simulink model "TosAeb"  (R2021a)
settings: Solver=FixedStepDiscrete  StartTime=0.0  StopTime=inf  FixedStep=0.01  RelTol=1e-3
undefined symbols (not defined in the file or in its folder): ACCModSts, AccSts1, AEB_ObjectMovingOrientation, AEB_ObjectMovingState, CN_BTCActive, CN_CurrentGearR, CN_CurrentGearR_DHT, CN_PTCActive, CN_VDCActive, CN_VSC_EspOff, CN_VSC_FCW_AEB_Error, CN_VSC_FCW_AEB_NoDispay, CN_VSC_FCW_AEB_PerfmDgdtn, CN_VSC_FCW_AEB_TempUnAvlbl, CN_VSC_VehStandstill, CNaccelAvoidMax, CNaccelAvoidMin, CNbrakePedalPress, CNcomfortStrLeftAccelMax, CNcomfortStrLeftAccelMin, CNcomfortStrRightAccelMax, CNcomfortStrRightAccelMin, CNDrvDoorOpen, CNDrvModeEco, CNDrvModeNorm, CNDrvModeOther, CNDrvModeSport, CNDrvSBRreminder, CNNull, CNobjInvalidID, CNPassDoorOpen, CNPassSBRreminder, CNPEBSAccelNegInf, CNPEBSAccelNull, CNPEBSTimeInf, CNPEBSTimeNull, CNSTOPMAX, CNSTOPNULL, CNsubjWidth, CNSwtOFF, CNSwtON, CNTTCMAX, CNTTCNULL, CNVehConfigAEBCycNone, CNVehConfigAEBNone, CNVehConfigAEBPedNone, CNVehConfigFCWNone, CNVehConfigJANone, CollisionSeverity, FALSE, FCWSnvty, JACycObjCalcInfoDefaultParams, JAPedObjCalcInfoDefaultParams, JAVehObjCalcInfoDefaultParams, latCycObjCalcInfoDefaultParams, latPedObjCalcInfoDefaultParams, longObjCalcInfoDefaultParams, ModSts, movingStatus, ObjectType ... (+1112)
libraries: simulink x3031, AEBSFunctionLib x50, Ta_Library x33, AEBSStateMachineLib x23, Mylibrary x12, simulink_extras x7, ObjSelect_lib x3, dspstat3 x2
(part 31 of 102)

== system TosAeb/FcwAebJa_Step_sys/ALG_AEBSystem/ALG_AEBController/AEB_StateMachineCalcApp/JA_AEBStateMachine/JA_pedAEBfullStateMachine/ActivationCondCalc ==
  JA_pedAEBfullSuppCond : Inport
  JA_pedAEBfullSuppArb : Inport  Port=2
  JA_pedAEBfullFuncImpSuppCond : Inport  Port=3
  Compare To Constant1 : simulink/Logic and Bit Operations/Compare To Constant  relop===  const=FALSE  OutDataTypeStr=boolean
  Compare To Constant2 : simulink/Logic and Bit Operations/Compare To Constant  relop===  const=FALSE  OutDataTypeStr=boolean
  Compare To Constant3 : simulink/Logic and Bit Operations/Compare To Constant  relop===  const=FALSE  OutDataTypeStr=boolean
  Logical Operator : Logic  Inputs=3  AllPortsSameDT=off  OutDataTypeStr=boolean
  ActivationCondFulfilled : Outport
  wires:
  JA_pedAEBfullSuppCond/1 -> Compare To Constant3/1
  JA_pedAEBfullSuppArb/1 -> Compare To Constant1/1
  JA_pedAEBfullFuncImpSuppCond/1 -> Compare To Constant2/1
  Compare To Constant1/1 -> Logical Operator/2
  Compare To Constant3/1 -> Logical Operator/1
  Compare To Constant2/1 -> Logical Operator/3
  Logical Operator/1 -> ActivationCondFulfilled/1

== system TosAeb/FcwAebJa_Step_sys/ALG_AEBSystem/ALG_AEBController/AEB_StateMachineCalcApp/JA_AEBStateMachine/JA_pedAEBfullStateMachine/ActiveStateAborCondCalc ==
  JA_pedAEBfullAborCond : Inport
  JA_pedAEBfullAborArb : Inport  Port=2
  JA_pedAEBfullFuncImpAborCond : Inport  Port=3
  JABrkManeuAborCond : Inport  Port=4
  Compare To Constant{,1..3} (4 alike) : simulink/Logic and Bit Operations/Compare To Constant  relop===  const=TRUE  OutDataTypeStr=boolean
  Logical Operator : Logic  Operator=OR  Inputs=4  AllPortsSameDT=off  OutDataTypeStr=boolean
  ActiveStateAborCondFulfilled : Outport
  wires:
  Logical Operator/1 -> ActiveStateAborCondFulfilled/1
  Compare To Constant2/1 -> Logical Operator/3
  Compare To Constant/1 -> Logical Operator/1
  Compare To Constant1/1 -> Logical Operator/2
  JA_pedAEBfullFuncImpAborCond/1 -> Compare To Constant2/1
  JA_pedAEBfullAborArb/1 -> Compare To Constant1/1
  JA_pedAEBfullAborCond/1 -> Compare To Constant/1
  JABrkManeuAborCond/1 -> Compare To Constant3/1
  Compare To Constant3/1 -> Logical Operator/4

== system TosAeb/FcwAebJa_Step_sys/ALG_AEBSystem/ALG_AEBController/AEB_StateMachineCalcApp/JA_AEBStateMachine/JA_pedAEBfullStateMachine/StandstillStateAborCondCalc ==
  JA_pedAEBfullAborCond : Inport
  JA_pedAEBfullAborArb : Inport  Port=2
  JA_pedAEBfullFuncImpAborCond : Inport  Port=3
  JABrkManeuAborCond : Inport  Port=4
  Compare To Constant{,1..3} (4 alike) : simulink/Logic and Bit Operations/Compare To Constant  relop===  const=TRUE  OutDataTypeStr=boolean
  Logical Operator : Logic  Operator=OR  Inputs=4  AllPortsSameDT=off  OutDataTypeStr=boolean
  ActiveStateAborCondFulfilled : Outport
  wires:
  Compare To Constant3/1 -> Logical Operator/4
  JABrkManeuAborCond/1 -> Compare To Constant3/1
  JA_pedAEBfullAborCond/1 -> Compare To Constant/1
  JA_pedAEBfullAborArb/1 -> Compare To Constant1/1
  JA_pedAEBfullFuncImpAborCond/1 -> Compare To Constant2/1
  Compare To Constant1/1 -> Logical Operator/2
  Compare To Constant/1 -> Logical Operator/1
  Compare To Constant2/1 -> Logical Operator/3
  Logical Operator/1 -> ActiveStateAborCondFulfilled/1

== system TosAeb/FcwAebJa_Step_sys/ALG_AEBSystem/ALG_AEBController/AEB_StateMachineCalcApp/JA_AEBStateMachine/JA_pedPrefillStateMachine/AEBFuncFaultMap ==
  FCW_AEB_VehFctSts : Inport
  Compare To Constant : simulink/Logic and Bit Operations/Compare To Constant  relop===  const=CN_VSC_FCW_AEB_Error  OutDataTypeStr=boolean
  Fault : Outport
  wires:
  FCW_AEB_VehFctSts/1 -> Compare To Constant/1
  Compare To Constant/1 -> Fault/1

== system TosAeb/FcwAebJa_Step_sys/ALG_AEBSystem/ALG_AEBController/AEB_StateMachineCalcApp/JA_AEBStateMachine/JA_pedPrefillStateMachine/ActivationCondCalc ==
  JA_pedPrefillSuppCond : Inport
  JA_pedPrefillSuppArb : Inport  Port=2
  JA_pedPrefillFuncImpSuppCond : Inport  Port=3
  Compare To Constant1 : simulink/Logic and Bit Operations/Compare To Constant  relop===  const=FALSE  OutDataTypeStr=boolean
  Compare To Constant2 : simulink/Logic and Bit Operations/Compare To Constant  relop===  const=FALSE  OutDataTypeStr=boolean
  Compare To Constant3 : simulink/Logic and Bit Operations/Compare To Constant  relop===  const=FALSE  OutDataTypeStr=boolean
  Logical Operator : Logic  Inputs=3  AllPortsSameDT=off  OutDataTypeStr=boolean
  ActivationCondFulfilled : Outport
  wires:
  JA_pedPrefillSuppCond/1 -> Compare To Constant3/1
  JA_pedPrefillSuppArb/1 -> Compare To Constant1/1
  JA_pedPrefillFuncImpSuppCond/1 -> Compare To Constant2/1
  Compare To Constant1/1 -> Logical Operator/2
  Compare To Constant3/1 -> Logical Operator/1
  Compare To Constant2/1 -> Logical Operator/3
  Logical Operator/1 -> ActivationCondFulfilled/1

== system TosAeb/FcwAebJa_Step_sys/ALG_AEBSystem/ALG_AEBController/AEB_StateMachineCalcApp/JA_AEBStateMachine/JA_pedPrefillStateMachine/ActiveStateAborCondCalc ==
  JA_pedPrefillAborCond : Inport
  JA_pedPrefillAborArb : Inport  Port=2
  JA_pedPrefillFuncImpAborCond : Inport  Port=3
  ABPManeuAborCond : Inport  Port=4
  Compare To Constant{,1..3} (4 alike) : simulink/Logic and Bit Operations/Compare To Constant  relop===  const=TRUE  OutDataTypeStr=boolean
  Logical Operator : Logic  Operator=OR  Inputs=4  AllPortsSameDT=off  OutDataTypeStr=boolean
  ActiveStateAborCondFulfilled : Outport
  wires:
  Logical Operator/1 -> ActiveStateAborCondFulfilled/1
  Compare To Constant2/1 -> Logical Operator/3
  Compare To Constant/1 -> Logical Operator/1
  Compare To Constant1/1 -> Logical Operator/2
  JA_pedPrefillFuncImpAborCond/1 -> Compare To Constant2/1
  JA_pedPrefillAborArb/1 -> Compare To Constant1/1
  JA_pedPrefillAborCond/1 -> Compare To Constant/1
  ABPManeuAborCond/1 -> Compare To Constant3/1
  Compare To Constant3/1 -> Logical Operator/4

== system TosAeb/FcwAebJa_Step_sys/ALG_AEBSystem/ALG_AEBController/AEB_StateMachineCalcApp/JA_AEBStateMachine/JA_vehAEBStateMachine/AEBFuncFaultMap ==
  FCW_AEB_VehFctSts : Inport
  Compare To Constant : simulink/Logic and Bit Operations/Compare To Constant  relop===  const=CN_VSC_FCW_AEB_Error  OutDataTypeStr=boolean
  Fault : Outport
  wires:
  FCW_AEB_VehFctSts/1 -> Compare To Constant/1
  Compare To Constant/1 -> Fault/1

== system TosAeb/FcwAebJa_Step_sys/ALG_AEBSystem/ALG_AEBController/AEB_StateMachineCalcApp/JA_AEBStateMachine/JA_vehAEBStateMachine/ActivationCondCalc ==
  JA_vehAEBfullSuppCond : Inport
  JA_vehAEBfullSuppArb : Inport  Port=2
  JA_vehAEBfullFuncImpSuppCond : Inport  Port=3
  Compare To Constant1 : simulink/Logic and Bit Operations/Compare To Constant  relop===  const=FALSE  OutDataTypeStr=boolean
  Compare To Constant2 : simulink/Logic and Bit Operations/Compare To Constant  relop===  const=FALSE  OutDataTypeStr=boolean
  Compare To Constant3 : simulink/Logic and Bit Operations/Compare To Constant  relop===  const=FALSE  OutDataTypeStr=boolean
  Logical Operator : Logic  Inputs=3  AllPortsSameDT=off  OutDataTypeStr=boolean
  ActivationCondFulfilled : Outport
  wires:
  JA_vehAEBfullSuppCond/1 -> Compare To Constant3/1
  JA_vehAEBfullSuppArb/1 -> Compare To Constant1/1
  JA_vehAEBfullFuncImpSuppCond/1 -> Compare To Constant2/1
  Compare To Constant1/1 -> Logical Operator/2
  Compare To Constant3/1 -> Logical Operator/1
  Compare To Constant2/1 -> Logical Operator/3
  Logical Operator/1 -> ActivationCondFulfilled/1

== system TosAeb/FcwAebJa_Step_sys/ALG_AEBSystem/ALG_AEBController/AEB_StateMachineCalcApp/JA_AEBStateMachine/JA_vehAEBStateMachine/ActiveStateAborCondCalc ==
  JA_vehAEBfullAborCondNoStandstill : Inport
  JA_vehAEBfullAborArb : Inport  Port=2
  JA_vehAEBfullFuncImpAborCond : Inport  Port=3
  JABrkManeuAborCond : Inport  Port=4
  Compare To Constant{,1..3} (4 alike) : simulink/Logic and Bit Operations/Compare To Constant  relop===  const=TRUE  OutDataTypeStr=boolean
  Logical Operator : Logic  Operator=OR  Inputs=4  AllPortsSameDT=off  OutDataTypeStr=boolean
  ActiveStateAborCondFulfilled : Outport
  wires:
  Logical Operator/1 -> ActiveStateAborCondFulfilled/1
  Compare To Constant2/1 -> Logical Operator/3
  Compare To Constant/1 -> Logical Operator/1
  Compare To Constant1/1 -> Logical Operator/2
  JA_vehAEBfullFuncImpAborCond/1 -> Compare To Constant2/1
  JA_vehAEBfullAborArb/1 -> Compare To Constant1/1
  JA_vehAEBfullAborCondNoStandstill/1 -> Compare To Constant/1
  JABrkManeuAborCond/1 -> Compare To Constant3/1
  Compare To Constant3/1 -> Logical Operator/4

== system TosAeb/FcwAebJa_Step_sys/ALG_AEBSystem/ALG_AEBController/AEB_StateMachineCalcApp/JA_AEBStateMachine/JA_vehAEBStateMachine/StandstillStateAborCondCalc ==
  JA_vehAEBfullAborCondStandstill : Inport
  JA_vehAEBfullAborArb : Inport  Port=2
  JA_vehAEBfullFuncImpAborCond : Inport  Port=3
  JABrkManeuAborCond : Inport  Port=4
  Compare To Constant{,1..3} (4 alike) : simulink/Logic and Bit Operations/Compare To Constant  relop===  const=TRUE  OutDataTypeStr=boolean
  Logical Operator : Logic  Operator=OR  Inputs=4  AllPortsSameDT=off  OutDataTypeStr=boolean
  ActiveStateAborCondFulfilled : Outport
  wires:
  Compare To Constant3/1 -> Logical Operator/4
  JABrkManeuAborCond/1 -> Compare To Constant3/1
  JA_vehAEBfullAborCondStandstill/1 -> Compare To Constant/1
  JA_vehAEBfullAborArb/1 -> Compare To Constant1/1
  JA_vehAEBfullFuncImpAborCond/1 -> Compare To Constant2/1
  Compare To Constant1/1 -> Logical Operator/2
  Compare To Constant/1 -> Logical Operator/1
  Compare To Constant2/1 -> Logical Operator/3
  Logical Operator/1 -> ActiveStateAborCondFulfilled/1

== system TosAeb/FcwAebJa_Step_sys/ALG_AEBSystem/ALG_AEBController/AEB_StateMachineCalcApp/JA_AEBStateMachine/JA_vehPrefillStateMachine/AEBFuncFaultMap ==
  FCW_AEB_VehFctSts : Inport
  Compare To Constant : simulink/Logic and Bit Operations/Compare To Constant  relop===  const=CN_VSC_FCW_AEB_Error  OutDataTypeStr=boolean
  Fault : Outport
  wires:
  FCW_AEB_VehFctSts/1 -> Compare To Constant/1
  Compare To Constant/1 -> Fault/1

== system TosAeb/FcwAebJa_Step_sys/ALG_AEBSystem/ALG_AEBController/AEB_StateMachineCalcApp/JA_AEBStateMachine/JA_vehPrefillStateMachine/ActivationCondCalc ==
  JA_vehPrefillSuppCond : Inport
  JA_vehPrefillSuppArb : Inport  Port=2
  JA_vehPrefillFuncImpSuppCond : Inport  Port=3
  Compare To Constant1 : simulink/Logic and Bit Operations/Compare To Constant  relop===  const=FALSE  OutDataTypeStr=boolean
  Compare To Constant2 : simulink/Logic and Bit Operations/Compare To Constant  relop===  const=FALSE  OutDataTypeStr=boolean
  Compare To Constant3 : simulink/Logic and Bit Operations/Compare To Constant  relop===  const=FALSE  OutDataTypeStr=boolean
  Logical Operator : Logic  Inputs=3  AllPortsSameDT=off  OutDataTypeStr=boolean
  ActivationCondFulfilled : Outport
  wires:
  JA_vehPrefillSuppCond/1 -> Compare To Constant3/1
  JA_vehPrefillSuppArb/1 -> Compare To Constant1/1
  JA_vehPrefillFuncImpSuppCond/1 -> Compare To Constant2/1
  Compare To Constant1/1 -> Logical Operator/2
  Compare To Constant3/1 -> Logical Operator/1
  Compare To Constant2/1 -> Logical Operator/3
  Logical Operator/1 -> ActivationCondFulfilled/1

== system TosAeb/FcwAebJa_Step_sys/ALG_AEBSystem/ALG_AEBController/AEB_StateMachineCalcApp/JA_AEBStateMachine/JA_vehPrefillStateMachine/ActiveStateAborCondCalc ==
  JA_vehPrefillAborCond : Inport
  JA_vehPrefillAborArb : Inport  Port=2
  JA_vehPrefillFuncImpAborCond : Inport  Port=3
  ABPManeuAborCond : Inport  Port=4
  Compare To Constant{,1..3} (4 alike) : simulink/Logic and Bit Operations/Compare To Constant  relop===  const=TRUE  OutDataTypeStr=boolean
  Logical Operator : Logic  Operator=OR  Inputs=4  AllPortsSameDT=off  OutDataTypeStr=boolean
  ActiveStateAborCondFulfilled : Outport
  wires:
  Logical Operator/1 -> ActiveStateAborCondFulfilled/1
  Compare To Constant2/1 -> Logical Operator/3
  Compare To Constant/1 -> Logical Operator/1
  Compare To Constant1/1 -> Logical Operator/2
  JA_vehPrefillFuncImpAborCond/1 -> Compare To Constant2/1
  JA_vehPrefillAborArb/1 -> Compare To Constant1/1
  JA_vehPrefillAborCond/1 -> Compare To Constant/1
  ABPManeuAborCond/1 -> Compare To Constant3/1
  Compare To Constant3/1 -> Logical Operator/4

== system TosAeb/FcwAebJa_Step_sys/ALG_AEBSystem/ALG_AEBController/AEB_StateMachineCalcApp/JaFctStsArb/BrkArb/BrkAborArb ==
  JA_vehAEBModSts : Inport
  JA_pedAEBModSts : Inport  Port=2
  JA_cycAEBModSts : Inport  Port=3
  JA_PTW_AEBModSts : Inport  Port=4
  Bus Creator : BusCreator  Inputs=4
  Bus Selector : BusSelector  OutputSignals=JA_pedAEBModSts,JA_cycAEBModSts,JA_PTW_AEBModSts
  Bus Selector1 : BusSelector  OutputSignals=JA_cycAEBModSts,JA_pedAEBModSts
  Bus Selector2 : BusSelector  OutputSignals=JA_pedAEBModSts
  Compare To Constant{1..2,4..6,12} (6 alike) : simulink/Logic and Bit Operations/Compare To Constant  relop===  const=ModSts.ActiveState  OutDataTypeStr=boolean
  Constant : Constant  Value=FALSE
  Logical Operator : Logic  Operator=OR  Inputs=3  AllPortsSameDT=off  OutDataTypeStr=boolean
  Logical Operator1 : Logic  Operator=OR  AllPortsSameDT=off  OutDataTypeStr=boolean
  JA_vehAEBAborArb : Outport
  JA_PTW_AEBAborArb : Outport  Port=2
  JA_cycAEBAborArb : Outport  Port=3
  JA_pedAEBAborArb : Outport  Port=4
  wires:
  JA_PTW_AEBModSts/1 -> Bus Creator/4  "JA_PTW_AEBModSts"
  JA_cycAEBModSts/1 -> Bus Creator/3  "JA_cycAEBModSts"
  JA_pedAEBModSts/1 -> Bus Creator/2  "JA_pedAEBModSts"
  JA_vehAEBModSts/1 -> Bus Creator/1  "JA_vehAEBModSts"
  Bus Selector/3 -> Compare To Constant1/1  "<JA_PTW_AEBModSts>"
  Bus Selector1/1 -> Compare To Constant5/1  "<JA_cycAEBModSts>"
  Bus Selector1/2 -> Compare To Constant4/1  "<JA_pedAEBModSts>"
  Compare To Constant2/1 -> Logical Operator/2
  Compare To Constant4/1 -> Logical Operator1/2
  Compare To Constant1/1 -> Logical Operator/3
  Bus Selector2/1 -> Compare To Constant12/1  "<JA_pedAEBModSts>"
  Bus Creator/1 -> Bus Selector2/1, Bus Selector1/1, Bus Selector/1
  Compare To Constant6/1 -> Logical Operator/1
  Bus Selector/1 -> Compare To Constant6/1  "<JA_pedAEBModSts>"
  Compare To Constant12/1 -> JA_cycAEBAborArb/1
  Bus Selector/2 -> Compare To Constant2/1  "<JA_cycAEBModSts>"
  Compare To Constant5/1 -> Logical Operator1/1
  Logical Operator/1 -> JA_vehAEBAborArb/1
  Logical Operator1/1 -> JA_PTW_AEBAborArb/1
  Constant/1 -> JA_pedAEBAborArb/1

== system TosAeb/FcwAebJa_Step_sys/ALG_AEBSystem/ALG_AEBController/AEB_StateMachineCalcApp/JaFctStsArb/BrkArb/JA_BrkActvArb ==
  JA_vehAEBModStsArb : Inport
  JA_PTW_AEBModStsArb : Inport  Port=2
  JA_cycAEBModStsArb : Inport  Port=3
  JA_pedAEBModStsArb : Inport  Port=4
  Compare To Constant{,1..3} (4 alike) : simulink/Logic and Bit Operations/Compare To Constant  relop===  const=ModSts.ActiveState  OutDataTypeStr=boolean
  Logical Operator : Logic  Operator=OR  Inputs=4  AllPortsSameDT=off  OutDataTypeStr=boolean
  JA_BrkActvCond : Outport
  wires:
  Compare To Constant2/1 -> Logical Operator/3
  Compare To Constant1/1 -> Logical Operator/2
  Compare To Constant/1 -> Logical Operator/1
  Logical Operator/1 -> JA_BrkActvCond/1
  JA_vehAEBModStsArb/1 -> Compare To Constant/1
  JA_PTW_AEBModStsArb/1 -> Compare To Constant1/1
  JA_cycAEBModStsArb/1 -> Compare To Constant2/1
  JA_pedAEBModStsArb/1 -> Compare To Constant3/1
  Compare To Constant3/1 -> Logical Operator/4

== system TosAeb/FcwAebJa_Step_sys/ALG_AEBSystem/ALG_AEBController/AEB_StateMachineCalcApp/JaFctStsArb/BrkArb/JA_BrkModStsArbtrtn ==
  JA_vehAEBModSts : Inport
  JA_PTW_AEBModSts : Inport  Port=2
  JA_cycAEBModSts : Inport  Port=3
  JA_pedAEBModSts : Inport  Port=4
  JA_vehAEBAborArb : Inport  Port=5
  JA_PTW_AEBAborArb : Inport  Port=6
  JA_cycAEBAborArb : Inport  Port=7
  JA_pedAEBAborArb : Inport  Port=8
  Compare To Constant{,2,4,6} (4 alike) : simulink/Logic and Bit Operations/Compare To Constant  relop=~=  const=ModSts.ActiveState  OutDataTypeStr=boolean
  Compare To Constant{1,3,5,7} (4 alike) : simulink/Logic and Bit Operations/Compare To Constant  relop===  const=FALSE  OutDataTypeStr=boolean
  Constant{,1..3} (4 alike) : Constant  Value=ModSts.StandbyState
  Switch{,1..7} (8 alike) : Switch  Criteria=u2 ~= 0
  JA_vehAEBModStsArb : Outport
  JA_PTW_AEBModStsArb : Outport  Port=2
  JA_cycAEBModStsArb : Outport  Port=3
  JA_pedAEBModStsArb : Outport  Port=4
  wires:
  Compare To Constant/1 -> Switch/2
  Switch1/1 -> Switch/3
  Compare To Constant1/1 -> Switch1/2
  JA_vehAEBAborArb/1 -> Compare To Constant1/1
  Constant/1 -> Switch1/3
  Switch/1 -> JA_vehAEBModStsArb/1
  Compare To Constant2/1 -> Switch2/2
  JA_PTW_AEBAborArb/1 -> Compare To Constant3/1
  Compare To Constant3/1 -> Switch3/2
  Constant1/1 -> Switch3/3
  Switch3/1 -> Switch2/3
  Switch2/1 -> JA_PTW_AEBModStsArb/1
  Switch4/1 -> JA_cycAEBModStsArb/1
  Switch5/1 -> Switch4/3
  Constant2/1 -> Switch5/3
  Compare To Constant5/1 -> Switch5/2
  JA_cycAEBAborArb/1 -> Compare To Constant5/1
  Compare To Constant4/1 -> Switch4/2
  JA_PTW_AEBModSts/1 -> Switch3/1, Switch2/1, Compare To Constant2/1  "JA_PTW_AEBModSts"
  JA_cycAEBModSts/1 -> Compare To Constant4/1, Switch4/1, Switch5/1  "JA_cycAEBModSts"
  JA_vehAEBModSts/1 -> Switch/1, Switch1/1, Compare To Constant/1  "JA_vehAEBModSts"
  JA_pedAEBModSts/1 -> Switch7/1, Switch6/1, Compare To Constant6/1
  Compare To Constant6/1 -> Switch6/2
  JA_pedAEBAborArb/1 -> Compare To Constant7/1
  Compare To Constant7/1 -> Switch7/2
  Constant3/1 -> Switch7/3
  Switch7/1 -> Switch6/3
  Switch6/1 -> JA_pedAEBModStsArb/1

== system TosAeb/FcwAebJa_Step_sys/ALG_AEBSystem/ALG_AEBController/AEB_StateMachineCalcApp/JaFctStsArb/BrkArb/JA_BrkSuppArb ==
  JA_vehAEBModSts : Inport
  JA_pedAEBModSts : Inport  Port=2
  JA_cycAEBModSts : Inport  Port=3
  JA_PTW_AEBModSts : Inport  Port=4
  Bus Creator : BusCreator  Inputs=4
  Bus Selector : BusSelector  OutputSignals=JA_pedAEBModSts,JA_cycAEBModSts,JA_PTW_AEBModSts
  Bus Selector1 : BusSelector  OutputSignals=JA_cycAEBModSts,JA_pedAEBModSts
  Bus Selector2 : BusSelector  OutputSignals=JA_cycAEBModSts
  Compare To Constant{1..2,4..6,12} (6 alike) : simulink/Logic and Bit Operations/Compare To Constant  relop===  const=ModSts.ActiveState  OutDataTypeStr=boolean
  Constant : Constant  Value=FALSE
  Logical Operator : Logic  Operator=OR  Inputs=3  AllPortsSameDT=off  OutDataTypeStr=boolean
  Logical Operator1 : Logic  Operator=OR  AllPortsSameDT=off  OutDataTypeStr=boolean
  JA_vehAEBSuppArb : Outport
  JA_PTW_AEBSuppArb : Outport  Port=2
  JA_cycAEBSuppArb : Outport  Port=3
  JA_pedAEBSuppArb : Outport  Port=4
  wires:
  Logical Operator/1 -> JA_vehAEBSuppArb/1
  Compare To Constant6/1 -> Logical Operator/1
  Compare To Constant2/1 -> Logical Operator/2
  Bus Selector/1 -> Compare To Constant6/1  "<JA_pedAEBModSts>"
  Bus Selector/2 -> Compare To Constant2/1  "<JA_cycAEBModSts>"
  Bus Selector/3 -> Compare To Constant1/1  "<JA_PTW_AEBModSts>"
  Compare To Constant1/1 -> Logical Operator/3
  Logical Operator1/1 -> JA_PTW_AEBSuppArb/1
  Compare To Constant12/1 -> JA_cycAEBSuppArb/1
  Constant/1 -> JA_pedAEBSuppArb/1
  JA_vehAEBModSts/1 -> Bus Creator/1  "JA_vehAEBModSts"
  JA_pedAEBModSts/1 -> Bus Creator/2  "JA_pedAEBModSts"
  JA_cycAEBModSts/1 -> Bus Creator/3  "JA_cycAEBModSts"
  JA_PTW_AEBModSts/1 -> Bus Creator/4  "JA_PTW_AEBModSts"
  Bus Creator/1 -> Bus Selector2/1, Bus Selector1/1, Bus Selector/1
  Bus Selector1/1 -> Compare To Constant5/1  "<JA_cycAEBModSts>"
  Bus Selector1/2 -> Compare To Constant4/1  "<JA_pedAEBModSts>"
  Compare To Constant5/1 -> Logical Operator1/1
  Compare To Constant4/1 -> Logical Operator1/2
  Bus Selector2/1 -> Compare To Constant12/1  "<JA_cycAEBModSts>"

== system TosAeb/FcwAebJa_Step_sys/ALG_AEBSystem/ALG_AEBController/AEB_StateMachineCalcApp/JaFctStsArb/BrkReadyArb/BrkAborArb ==
  JA_vehPrefillModSts : Inport
  JA_pedPrefillModSts : Inport  Port=2
  JA_cycPrefillModSts : Inport  Port=3
  JA_PTW_PrefillModSts : Inport  Port=4
  JA_BrkActvCond : Inport  Port=5
  Bus Creator : BusCreator  Inputs=5
  Bus Selector : BusSelector  OutputSignals=JA_pedAEBModSts,JA_cycAEBModSts,JA_PTW_AEBModSts
  Bus Selector1 : BusSelector  OutputSignals=JA_cycAEBModSts,JA_pedAEBModSts
  Bus Selector2 : BusSelector  OutputSignals=JA_pedAEBModSts
  Compare To Constant{1..2,4..6,12} (6 alike) : simulink/Logic and Bit Operations/Compare To Constant  relop===  const=ModSts.ActiveState  OutDataTypeStr=boolean
  Constant : Constant  Value=FALSE
  Logical Operator : Logic  Operator=OR  Inputs=3  AllPortsSameDT=off  OutDataTypeStr=boolean
  Logical Operator1 : Logic  Operator=OR  AllPortsSameDT=off  OutDataTypeStr=boolean
  JA_vehPrefillAborArb : Outport
  JA_PTW_PrefillAborArb : Outport  Port=2
  JA_cycPrefillAborArb : Outport  Port=3
  JA_pedPrefillAborArb : Outport  Port=4
  wires:
  Constant/1 -> JA_pedPrefillAborArb/1
  Logical Operator1/1 -> JA_PTW_PrefillAborArb/1
  Logical Operator/1 -> JA_vehPrefillAborArb/1
  Compare To Constant5/1 -> Logical Operator1/1
  Bus Selector/2 -> Compare To Constant2/1  "<JA_cycAEBModSts>"
  Compare To Constant12/1 -> JA_cycPrefillAborArb/1
  Bus Selector/1 -> Compare To Constant6/1  "<JA_pedAEBModSts>"
  Compare To Constant6/1 -> Logical Operator/1
  Bus Creator/1 -> Bus Selector/1, Bus Selector1/1, Bus Selector2/1
  Bus Selector2/1 -> Compare To Constant12/1  "<JA_pedAEBModSts>"
  Compare To Constant1/1 -> Logical Operator/3
  Compare To Constant4/1 -> Logical Operator1/2
  Compare To Constant2/1 -> Logical Operator/2
  Bus Selector1/2 -> Compare To Constant4/1  "<JA_pedAEBModSts>"
  Bus Selector1/1 -> Compare To Constant5/1  "<JA_cycAEBModSts>"
  Bus Selector/3 -> Compare To Constant1/1  "<JA_PTW_AEBModSts>"
  JA_vehPrefillModSts/1 -> Bus Creator/1  "JA_vehAEBModSts"
  JA_BrkActvCond/1 -> Bus Creator/5  "JA_BrkActvCond"
  JA_pedPrefillModSts/1 -> Bus Creator/2  "JA_pedAEBModSts"
  JA_cycPrefillModSts/1 -> Bus Creator/3  "JA_cycAEBModSts"
  JA_PTW_PrefillModSts/1 -> Bus Creator/4  "JA_PTW_AEBModSts"

== system TosAeb/FcwAebJa_Step_sys/ALG_AEBSystem/ALG_AEBController/AEB_StateMachineCalcApp/JaFctStsArb/BrkReadyArb/BrkReadyActvArb ==
  JA_vehPrefillModStsArb : Inport
  JA_PTW_PrefillModStsArb : Inport  Port=2
  JA_cycPrefillModStsArb : Inport  Port=3
  JA_pedPrefillModStsArb : Inport  Port=4
  Compare To Constant{,1..3} (4 alike) : simulink/Logic and Bit Operations/Compare To Constant  relop===  const=ModSts.ActiveState  OutDataTypeStr=boolean
  Logical Operator : Logic  Operator=OR  Inputs=4  AllPortsSameDT=off  OutDataTypeStr=boolean
  JA_BrkActvCond : Outport
  wires:
  Compare To Constant3/1 -> Logical Operator/4
  JA_pedPrefillModStsArb/1 -> Compare To Constant3/1
  JA_cycPrefillModStsArb/1 -> Compare To Constant2/1
  JA_PTW_PrefillModStsArb/1 -> Compare To Constant1/1
  JA_vehPrefillModStsArb/1 -> Compare To Constant/1
  Logical Operator/1 -> JA_BrkActvCond/1
  Compare To Constant/1 -> Logical Operator/1
  Compare To Constant1/1 -> Logical Operator/2
  Compare To Constant2/1 -> Logical Operator/3

== system TosAeb/FcwAebJa_Step_sys/ALG_AEBSystem/ALG_AEBController/AEB_StateMachineCalcApp/JaFctStsArb/BrkReadyArb/BrkReadyModStsArbtrtn ==
  JA_vehPrefillModSts : Inport
  JA_PTW_PrefillModSts : Inport  Port=2
  JA_cycPrefillModSts : Inport  Port=3
  JA_pedPrefillModSts : Inport  Port=4
  JA_vehPrefillAborArb : Inport  Port=5
  JA_PTW_PrefillAborArb : Inport  Port=6
  JA_cycPrefillAborArb : Inport  Port=7
  JA_pedPrefillAborArb : Inport  Port=8
  Compare To Constant{,2,4,6} (4 alike) : simulink/Logic and Bit Operations/Compare To Constant  relop=~=  const=ModSts.ActiveState  OutDataTypeStr=boolean
  Compare To Constant{1,3,5,7} (4 alike) : simulink/Logic and Bit Operations/Compare To Constant  relop===  const=FALSE  OutDataTypeStr=boolean
  Constant{,1..3} (4 alike) : Constant  Value=ModSts.StandbyState
  Switch{,1..7} (8 alike) : Switch  Criteria=u2 ~= 0
  JA_vehPrefillModStsArb : Outport
  JA_PTW_PrefillModStsArb : Outport  Port=2
  JA_cycPrefillModStsArb : Outport  Port=3
  JA_pedPrefillModStsArb : Outport  Port=4
  wires:
  Switch6/1 -> JA_pedPrefillModStsArb/1
  Switch7/1 -> Switch6/3
  Constant3/1 -> Switch7/3
  Compare To Constant7/1 -> Switch7/2
  JA_pedPrefillAborArb/1 -> Compare To Constant7/1
  Compare To Constant6/1 -> Switch6/2
  JA_pedPrefillModSts/1 -> Compare To Constant6/1, Switch6/1, Switch7/1  "JA_cycAEBModSts"
  JA_vehPrefillModSts/1 -> Compare To Constant/1, Switch1/1, Switch/1  "JA_vehAEBModSts"
  JA_cycPrefillModSts/1 -> Switch5/1, Switch4/1, Compare To Constant4/1  "JA_cycAEBModSts"
  JA_PTW_PrefillModSts/1 -> Compare To Constant2/1, Switch2/1, Switch3/1  "JA_PTW_AEBModSts"
  Compare To Constant4/1 -> Switch4/2
  JA_cycPrefillAborArb/1 -> Compare To Constant5/1
  Compare To Constant5/1 -> Switch5/2
  Constant2/1 -> Switch5/3
  Switch5/1 -> Switch4/3
  Switch4/1 -> JA_cycPrefillModStsArb/1
  Switch2/1 -> JA_PTW_PrefillModStsArb/1
  Switch3/1 -> Switch2/3
  Constant1/1 -> Switch3/3
  Compare To Constant3/1 -> Switch3/2
  JA_PTW_PrefillAborArb/1 -> Compare To Constant3/1
  Compare To Constant2/1 -> Switch2/2
  Switch/1 -> JA_vehPrefillModStsArb/1
  Constant/1 -> Switch1/3
  JA_vehPrefillAborArb/1 -> Compare To Constant1/1
  Compare To Constant1/1 -> Switch1/2
  Switch1/1 -> Switch/3
  Compare To Constant/1 -> Switch/2

== system TosAeb/FcwAebJa_Step_sys/ALG_AEBSystem/ALG_AEBController/AEB_StateMachineCalcApp/JaFctStsArb/BrkReadyArb/BrkReadySuppArb ==
  JA_vehPrefillModSts : Inport
  JA_pedPrefillModSts : Inport  Port=2
  JA_cycPrefillModSts : Inport  Port=3
  JA_PTW_PrefillModSts : Inport  Port=4
  JA_BrkActvCond : Inport  Port=5
  Bus Creator : BusCreator  Inputs=5
  Bus Selector : BusSelector  OutputSignals=JA_pedAEBModSts,JA_cycAEBModSts,JA_PTW_AEBModSts,JA_BrkActvCond
  Bus Selector1 : BusSelector  OutputSignals=JA_cycAEBModSts,JA_pedAEBModSts,JA_BrkActvCond
  Bus Selector2 : BusSelector  OutputSignals=JA_cycAEBModSts,JA_BrkActvCond
  Bus Selector3 : BusSelector  OutputSignals=JA_BrkActvCond
  Compare To Constant{1..2,4..6,12} (6 alike) : simulink/Logic and Bit Operations/Compare To Constant  relop===  const=ModSts.ActiveState  OutDataTypeStr=boolean
  Compare To Constant{3,7,11,14} (4 alike) : simulink/Logic and Bit Operations/Compare To Constant  relop===  const=TRUE  OutDataTypeStr=boolean
  Logical Operator : Logic  Operator=OR  Inputs=4  AllPortsSameDT=off  OutDataTypeStr=boolean
  Logical Operator1 : Logic  Operator=OR  Inputs=3  AllPortsSameDT=off  OutDataTypeStr=boolean
  Logical Operator2 : Logic  Operator=OR  AllPortsSameDT=off  OutDataTypeStr=boolean
  JA_vehPrefillSuppArb : Outport
  JA_PTW_PrefillSuppArb : Outport  Port=2
  JA_cycPrefillSuppArb : Outport  Port=3
  JA_pedPrefillSuppArb : Outport  Port=4
  wires:
  Bus Selector3/1 -> Compare To Constant14/1  "<JA_BrkActvCond>"
  Bus Selector2/2 -> Compare To Constant11/1  "<JA_BrkActvCond>"
  Bus Selector2/1 -> Compare To Constant12/1  "<JA_cycAEBModSts>"
  Compare To Constant7/1 -> Logical Operator1/3
  Compare To Constant4/1 -> Logical Operator1/2
  Compare To Constant5/1 -> Logical Operator1/1
  Bus Selector1/3 -> Compare To Constant7/1  "<JA_BrkActvCond>"
  Bus Selector1/2 -> Compare To Constant4/1  "<JA_pedAEBModSts>"
  Bus Selector1/1 -> Compare To Constant5/1  "<JA_cycAEBModSts>"
  Bus Selector/4 -> Compare To Constant3/1  "<JA_BrkActvCond>"
  Bus Creator/1 -> Bus Selector/1, Bus Selector1/1, Bus Selector2/1, Bus Selector3/1
  JA_BrkActvCond/1 -> Bus Creator/5  "JA_BrkActvCond"
  JA_PTW_PrefillModSts/1 -> Bus Creator/4  "JA_PTW_AEBModSts"
  JA_cycPrefillModSts/1 -> Bus Creator/3  "JA_cycAEBModSts"
  JA_pedPrefillModSts/1 -> Bus Creator/2  "JA_pedAEBModSts"
  JA_vehPrefillModSts/1 -> Bus Creator/1  "JA_vehAEBModSts"
  Compare To Constant14/1 -> JA_pedPrefillSuppArb/1
  Compare To Constant11/1 -> Logical Operator2/2
  Compare To Constant12/1 -> Logical Operator2/1
  Logical Operator2/1 -> JA_cycPrefillSuppArb/1
  Logical Operator1/1 -> JA_PTW_PrefillSuppArb/1
  Compare To Constant3/1 -> Logical Operator/4
  Compare To Constant1/1 -> Logical Operator/3
  Bus Selector/3 -> Compare To Constant1/1  "<JA_PTW_AEBModSts>"
  Bus Selector/2 -> Compare To Constant2/1  "<JA_cycAEBModSts>"
  Bus Selector/1 -> Compare To Constant6/1  "<JA_pedAEBModSts>"
  Compare To Constant2/1 -> Logical Operator/2
  Compare To Constant6/1 -> Logical Operator/1
  Logical Operator/1 -> JA_vehPrefillSuppArb/1

== system TosAeb/FcwAebJa_Step_sys/ALG_AEBSystem/ALG_AEBController/AEB_StateMachineCalcApp/LatAEBStateMachine/cycAEBfullStateMachine/AEBFuncFaultMap ==
  FCW_AEB_VehFctSts : Inport
  Compare To Constant : simulink/Logic and Bit Operations/Compare To Constant  relop===  const=CN_VSC_FCW_AEB_Error  OutDataTypeStr=boolean
  Fault : Outport
  wires:
  FCW_AEB_VehFctSts/1 -> Compare To Constant/1
  Compare To Constant/1 -> Fault/1

== system TosAeb/FcwAebJa_Step_sys/ALG_AEBSystem/ALG_AEBController/AEB_StateMachineCalcApp/LatAEBStateMachine/cycAEBfullStateMachine/ActivationCondCalc ==
  cycAEBfullSuppCond : Inport
  cycAEBfullSuppArb : Inport  Port=2
  cycAEBfullFuncImpSuppCond : Inport  Port=3
  Compare To Constant1 : simulink/Logic and Bit Operations/Compare To Constant  relop===  const=FALSE  OutDataTypeStr=boolean
  Compare To Constant2 : simulink/Logic and Bit Operations/Compare To Constant  relop===  const=FALSE  OutDataTypeStr=boolean
  Compare To Constant3 : simulink/Logic and Bit Operations/Compare To Constant  relop===  const=FALSE  OutDataTypeStr=boolean
  Logical Operator : Logic  Inputs=3  AllPortsSameDT=off  OutDataTypeStr=boolean
  ActivationCondFulfilled : Outport
  wires:
  cycAEBfullSuppCond/1 -> Compare To Constant3/1
  cycAEBfullSuppArb/1 -> Compare To Constant1/1
  cycAEBfullFuncImpSuppCond/1 -> Compare To Constant2/1
  Compare To Constant1/1 -> Logical Operator/2
  Compare To Constant3/1 -> Logical Operator/1
  Compare To Constant2/1 -> Logical Operator/3
  Logical Operator/1 -> ActivationCondFulfilled/1

== system TosAeb/FcwAebJa_Step_sys/ALG_AEBSystem/ALG_AEBController/AEB_StateMachineCalcApp/LatAEBStateMachine/cycAEBfullStateMachine/ActiveStateAborCondCalc ==
  cycAEBfullAborCondNoStandstill : Inport
  cycAEBfullAborArb : Inport  Port=2
  cycAEBfullFuncImpAborCond : Inport  Port=3
  latBrkManeuAborCond : Inport  Port=4
  Compare To Constant{,1..3} (4 alike) : simulink/Logic and Bit Operations/Compare To Constant  relop===  const=TRUE  OutDataTypeStr=boolean
  Logical Operator : Logic  Operator=OR  Inputs=4  AllPortsSameDT=off  OutDataTypeStr=boolean
  ActiveStateAborCondFulfilled : Outport
  wires:
  Logical Operator/1 -> ActiveStateAborCondFulfilled/1
  Compare To Constant2/1 -> Logical Operator/3
  Compare To Constant/1 -> Logical Operator/1
  Compare To Constant1/1 -> Logical Operator/2
  cycAEBfullFuncImpAborCond/1 -> Compare To Constant2/1
  cycAEBfullAborArb/1 -> Compare To Constant1/1
  cycAEBfullAborCondNoStandstill/1 -> Compare To Constant/1
  latBrkManeuAborCond/1 -> Compare To Constant3/1
  Compare To Constant3/1 -> Logical Operator/4

== system TosAeb/FcwAebJa_Step_sys/ALG_AEBSystem/ALG_AEBController/AEB_StateMachineCalcApp/LatAEBStateMachine/cycAEBfullStateMachine/StandstillAborCondCalc ==
  cycAEBfullAborCondStandstill : Inport
  cycAEBfullAborArb : Inport  Port=2
  cycAEBfullFuncImpAborCond : Inport  Port=3
  latBrkManeuAborCond : Inport  Port=4
  Compare To Constant{,1..3} (4 alike) : simulink/Logic and Bit Operations/Compare To Constant  relop===  const=TRUE  OutDataTypeStr=boolean
  Logical Operator : Logic  Operator=OR  Inputs=4  AllPortsSameDT=off  OutDataTypeStr=boolean
  ActiveStateAborCondFulfilled : Outport
  wires:
  Compare To Constant3/1 -> Logical Operator/4
  latBrkManeuAborCond/1 -> Compare To Constant3/1
  cycAEBfullAborCondStandstill/1 -> Compare To Constant/1
  cycAEBfullAborArb/1 -> Compare To Constant1/1
  cycAEBfullFuncImpAborCond/1 -> Compare To Constant2/1
  Compare To Constant1/1 -> Logical Operator/2
  Compare To Constant/1 -> Logical Operator/1
  Compare To Constant2/1 -> Logical Operator/3
  Logical Operator/1 -> ActiveStateAborCondFulfilled/1

== system TosAeb/FcwAebJa_Step_sys/ALG_AEBSystem/ALG_AEBController/AEB_StateMachineCalcApp/LatAEBStateMachine/cycAEBpartStateMachine/AEBFuncFaultMap ==
  FCW_AEB_VehFctSts : Inport
  Compare To Constant : simulink/Logic and Bit Operations/Compare To Constant  relop===  const=CN_VSC_FCW_AEB_Error  OutDataTypeStr=boolean
  Fault : Outport
  wires:
  FCW_AEB_VehFctSts/1 -> Compare To Constant/1
  Compare To Constant/1 -> Fault/1

== system TosAeb/FcwAebJa_Step_sys/ALG_AEBSystem/ALG_AEBController/AEB_StateMachineCalcApp/LatAEBStateMachine/cycAEBpartStateMachine/ActivationCondCalc ==
  cycAEBpartSuppCond : Inport
  cycAEBpartSuppArb : Inport  Port=2
  cycAEBpartFuncImpSuppCond : Inport  Port=3
  Compare To Constant1 : simulink/Logic and Bit Operations/Compare To Constant  relop===  const=FALSE  OutDataTypeStr=boolean
  Compare To Constant2 : simulink/Logic and Bit Operations/Compare To Constant  relop===  const=FALSE  OutDataTypeStr=boolean
  Compare To Constant3 : simulink/Logic and Bit Operations/Compare To Constant  relop===  const=FALSE  OutDataTypeStr=boolean
  Logical Operator : Logic  Inputs=3  AllPortsSameDT=off  OutDataTypeStr=boolean
  ActivationCondFulfilled : Outport
  wires:
  cycAEBpartSuppCond/1 -> Compare To Constant3/1
  cycAEBpartSuppArb/1 -> Compare To Constant1/1
  cycAEBpartFuncImpSuppCond/1 -> Compare To Constant2/1
  Compare To Constant1/1 -> Logical Operator/2
  Compare To Constant3/1 -> Logical Operator/1
  Compare To Constant2/1 -> Logical Operator/3
  Logical Operator/1 -> ActivationCondFulfilled/1

== system TosAeb/FcwAebJa_Step_sys/ALG_AEBSystem/ALG_AEBController/AEB_StateMachineCalcApp/LatAEBStateMachine/cycAEBpartStateMachine/ActiveStateAborCondCalc ==
  cycAEBpartAborCondNoStandstill : Inport
  cycAEBpartAborArb : Inport  Port=2
  cycAEBpartFuncImpAborCond : Inport  Port=3
  latBrkManeuAborCond : Inport  Port=4
  Compare To Constant{,1..3} (4 alike) : simulink/Logic and Bit Operations/Compare To Constant  relop===  const=TRUE  OutDataTypeStr=boolean
  Logical Operator : Logic  Operator=OR  Inputs=4  AllPortsSameDT=off  OutDataTypeStr=boolean
  ActiveStateAborCondFulfilled : Outport
  wires:
  Logical Operator/1 -> ActiveStateAborCondFulfilled/1
  Compare To Constant2/1 -> Logical Operator/3
  Compare To Constant/1 -> Logical Operator/1
  Compare To Constant1/1 -> Logical Operator/2
  cycAEBpartFuncImpAborCond/1 -> Compare To Constant2/1
  cycAEBpartAborArb/1 -> Compare To Constant1/1
  cycAEBpartAborCondNoStandstill/1 -> Compare To Constant/1
  latBrkManeuAborCond/1 -> Compare To Constant3/1
  Compare To Constant3/1 -> Logical Operator/4

== system TosAeb/FcwAebJa_Step_sys/ALG_AEBSystem/ALG_AEBController/AEB_StateMachineCalcApp/LatAEBStateMachine/cycAEBpartStateMachine/StandstillAborCondCalc ==
  cycAEBpartAborCondStandstill : Inport
  cycAEBpartAborArb : Inport  Port=2
  cycAEBpartFuncImpAborCond : Inport  Port=3
  latBrkManeuAborCond : Inport  Port=4
  Compare To Constant{,1..3} (4 alike) : simulink/Logic and Bit Operations/Compare To Constant  relop===  const=TRUE  OutDataTypeStr=boolean
  Logical Operator : Logic  Operator=OR  Inputs=4  AllPortsSameDT=off  OutDataTypeStr=boolean
  ActiveStateAborCondFulfilled : Outport
  wires:
  Compare To Constant3/1 -> Logical Operator/4
  latBrkManeuAborCond/1 -> Compare To Constant3/1
  cycAEBpartAborCondStandstill/1 -> Compare To Constant/1
  cycAEBpartAborArb/1 -> Compare To Constant1/1
  cycAEBpartFuncImpAborCond/1 -> Compare To Constant2/1
  Compare To Constant1/1 -> Logical Operator/2
  Compare To Constant/1 -> Logical Operator/1
  Compare To Constant2/1 -> Logical Operator/3
  Logical Operator/1 -> ActiveStateAborCondFulfilled/1

== system TosAeb/FcwAebJa_Step_sys/ALG_AEBSystem/ALG_AEBController/AEB_StateMachineCalcApp/LatAEBStateMachine/cycPrefillStateMachine/AEBFuncFaultMap ==
  FCW_AEB_VehFctSts : Inport
  Compare To Constant : simulink/Logic and Bit Operations/Compare To Constant  relop===  const=CN_VSC_FCW_AEB_Error  OutDataTypeStr=boolean
  Fault : Outport
  wires:
  FCW_AEB_VehFctSts/1 -> Compare To Constant/1
  Compare To Constant/1 -> Fault/1

== system TosAeb/FcwAebJa_Step_sys/ALG_AEBSystem/ALG_AEBController/AEB_StateMachineCalcApp/LatAEBStateMachine/cycPrefillStateMachine/ActivationCondCalc ==
  cycPrefillSuppCond : Inport
  cycPrefillSuppArb : Inport  Port=2
  cycPrefillFuncImpSuppCond : Inport  Port=3
  Compare To Constant1 : simulink/Logic and Bit Operations/Compare To Constant  relop===  const=FALSE  OutDataTypeStr=boolean
  Compare To Constant2 : simulink/Logic and Bit Operations/Compare To Constant  relop===  const=FALSE  OutDataTypeStr=boolean
  Compare To Constant3 : simulink/Logic and Bit Operations/Compare To Constant  relop===  const=FALSE  OutDataTypeStr=boolean
  Logical Operator : Logic  Inputs=3  AllPortsSameDT=off  OutDataTypeStr=boolean
  ActivationCondFulfilled : Outport
  wires:
  cycPrefillSuppCond/1 -> Compare To Constant3/1
  cycPrefillSuppArb/1 -> Compare To Constant1/1
  cycPrefillFuncImpSuppCond/1 -> Compare To Constant2/1
  Compare To Constant1/1 -> Logical Operator/2
  Compare To Constant3/1 -> Logical Operator/1
  Compare To Constant2/1 -> Logical Operator/3
  Logical Operator/1 -> ActivationCondFulfilled/1

== system TosAeb/FcwAebJa_Step_sys/ALG_AEBSystem/ALG_AEBController/AEB_StateMachineCalcApp/LatAEBStateMachine/cycPrefillStateMachine/ActiveStateAborCondCalc ==
  cycPrefillAborCond : Inport
  cycPrefillAborArb : Inport  Port=2
  cycPrefillFuncImpAborCond : Inport  Port=3
  ABPFuncManeuAborCond : Inport  Port=4
  Compare To Constant{,1..3} (4 alike) : simulink/Logic and Bit Operations/Compare To Constant  relop===  const=TRUE  OutDataTypeStr=boolean
  Logical Operator : Logic  Operator=OR  Inputs=4  AllPortsSameDT=off  OutDataTypeStr=boolean
  ActiveStateAborCondFulfilled : Outport
  wires:
  Logical Operator/1 -> ActiveStateAborCondFulfilled/1
  Compare To Constant2/1 -> Logical Operator/3
  Compare To Constant/1 -> Logical Operator/1
  Compare To Constant1/1 -> Logical Operator/2
  cycPrefillFuncImpAborCond/1 -> Compare To Constant2/1
  cycPrefillAborArb/1 -> Compare To Constant1/1
  cycPrefillAborCond/1 -> Compare To Constant/1
  ABPFuncManeuAborCond/1 -> Compare To Constant3/1
  Compare To Constant3/1 -> Logical Operator/4

== system TosAeb/FcwAebJa_Step_sys/ALG_AEBSystem/ALG_AEBController/AEB_StateMachineCalcApp/LatAEBStateMachine/pedABAStateMachine/AEBFuncFaultMap ==
  FCW_AEB_VehFctSts : Inport
  Compare To Constant : simulink/Logic and Bit Operations/Compare To Constant  relop===  const=CN_VSC_FCW_AEB_Error  OutDataTypeStr=boolean
  Fault : Outport
  wires:
  FCW_AEB_VehFctSts/1 -> Compare To Constant/1
  Compare To Constant/1 -> Fault/1

== system TosAeb/FcwAebJa_Step_sys/ALG_AEBSystem/ALG_AEBController/AEB_StateMachineCalcApp/LatAEBStateMachine/pedABAStateMachine/ActivationCondCalc ==
  pedABASuppCond : Inport
  pedABASuppArb : Inport  Port=2
  pedABAFuncImpSuppCond : Inport  Port=3
  Compare To Constant : simulink/Logic and Bit Operations/Compare To Constant  relop===  const=FALSE  OutDataTypeStr=boolean
  Compare To Constant1 : simulink/Logic and Bit Operations/Compare To Constant  relop===  const=FALSE  OutDataTypeStr=boolean
  Compare To Constant2 : simulink/Logic and Bit Operations/Compare To Constant  relop===  const=FALSE  OutDataTypeStr=boolean
  Logical Operator : Logic  Inputs=3  AllPortsSameDT=off  OutDataTypeStr=boolean
  ActivationCondFulfilled : Outport
  wires:
  pedABASuppCond/1 -> Compare To Constant/1
  pedABASuppArb/1 -> Compare To Constant1/1
  pedABAFuncImpSuppCond/1 -> Compare To Constant2/1
  Compare To Constant1/1 -> Logical Operator/2
  Compare To Constant/1 -> Logical Operator/1
  Compare To Constant2/1 -> Logical Operator/3
  Logical Operator/1 -> ActivationCondFulfilled/1

== system TosAeb/FcwAebJa_Step_sys/ALG_AEBSystem/ALG_AEBController/AEB_StateMachineCalcApp/LatAEBStateMachine/pedABAStateMachine/ActiveStateAborCondCalc ==
  pedABAAborCond : Inport
  pedABAAborArb : Inport  Port=2
  pedABAFuncImpAborCond : Inport  Port=3
  ABAFuncManeuAborCond : Inport  Port=4
  Compare To Constant{,1..3} (4 alike) : simulink/Logic and Bit Operations/Compare To Constant  relop===  const=TRUE  OutDataTypeStr=boolean
  Logical Operator : Logic  Operator=OR  Inputs=4  AllPortsSameDT=off  OutDataTypeStr=boolean
  ActiveStateAborCondFulfilled : Outport
  wires:
  Logical Operator/1 -> ActiveStateAborCondFulfilled/1
  Compare To Constant2/1 -> Logical Operator/3
  Compare To Constant/1 -> Logical Operator/1
  Compare To Constant1/1 -> Logical Operator/2
  pedABAFuncImpAborCond/1 -> Compare To Constant2/1
  pedABAAborArb/1 -> Compare To Constant1/1
  pedABAAborCond/1 -> Compare To Constant/1
  ABAFuncManeuAborCond/1 -> Compare To Constant3/1
  Compare To Constant3/1 -> Logical Operator/4

== system TosAeb/FcwAebJa_Step_sys/ALG_AEBSystem/ALG_AEBController/AEB_StateMachineCalcApp/LatAEBStateMachine/pedAEBfullStateMachine/AEBFuncFaultMap ==
  FCW_AEB_VehFctSts : Inport
  Compare To Constant : simulink/Logic and Bit Operations/Compare To Constant  relop===  const=CN_VSC_FCW_AEB_Error  OutDataTypeStr=boolean
  Fault : Outport
  wires:
  FCW_AEB_VehFctSts/1 -> Compare To Constant/1
  Compare To Constant/1 -> Fault/1

== system TosAeb/FcwAebJa_Step_sys/ALG_AEBSystem/ALG_AEBController/AEB_StateMachineCalcApp/LatAEBStateMachine/pedAEBfullStateMachine/ActivationCondCalc ==
  pedAEBfullSuppCond : Inport
  pedAEBfullSuppArb : Inport  Port=2
  pedAEBfullFuncImpSuppCond : Inport  Port=3
  Compare To Constant : simulink/Logic and Bit Operations/Compare To Constant  relop===  const=FALSE  OutDataTypeStr=boolean
  Compare To Constant1 : simulink/Logic and Bit Operations/Compare To Constant  relop===  const=FALSE  OutDataTypeStr=boolean
  Compare To Constant2 : simulink/Logic and Bit Operations/Compare To Constant  relop===  const=FALSE  OutDataTypeStr=boolean
  Logical Operator : Logic  Inputs=3  AllPortsSameDT=off  OutDataTypeStr=boolean
  ActivationCondFulfilled : Outport
  wires:
  pedAEBfullSuppCond/1 -> Compare To Constant/1
  pedAEBfullSuppArb/1 -> Compare To Constant1/1
  pedAEBfullFuncImpSuppCond/1 -> Compare To Constant2/1
  Compare To Constant1/1 -> Logical Operator/2
  Compare To Constant/1 -> Logical Operator/1
  Compare To Constant2/1 -> Logical Operator/3
  Logical Operator/1 -> ActivationCondFulfilled/1

== system TosAeb/FcwAebJa_Step_sys/ALG_AEBSystem/ALG_AEBController/AEB_StateMachineCalcApp/LatAEBStateMachine/pedAEBfullStateMachine/ActiveStateAborCondCalc ==
  pedAEBfullAborCondNoStandstill : Inport
  pedAEBfullAborArb : Inport  Port=2
  pedAEBfullFuncImpAborCond : Inport  Port=3
  latBrkManeuAborCond : Inport  Port=4
  Compare To Constant{,1..3} (4 alike) : simulink/Logic and Bit Operations/Compare To Constant  relop===  const=TRUE  OutDataTypeStr=boolean
  Logical Operator : Logic  Operator=OR  Inputs=4  AllPortsSameDT=off  OutDataTypeStr=boolean
  ActiveStateAborCondFulfilled : Outport
  wires:
  Logical Operator/1 -> ActiveStateAborCondFulfilled/1
  Compare To Constant2/1 -> Logical Operator/3
  Compare To Constant/1 -> Logical Operator/1
  Compare To Constant1/1 -> Logical Operator/2
  pedAEBfullFuncImpAborCond/1 -> Compare To Constant2/1
  pedAEBfullAborArb/1 -> Compare To Constant1/1
  pedAEBfullAborCondNoStandstill/1 -> Compare To Constant/1
  latBrkManeuAborCond/1 -> Compare To Constant3/1
  Compare To Constant3/1 -> Logical Operator/4

== system TosAeb/FcwAebJa_Step_sys/ALG_AEBSystem/ALG_AEBController/AEB_StateMachineCalcApp/LatAEBStateMachine/pedAEBfullStateMachine/StandstillAborCondCalc ==
  pedAEBfullAborCondStandstill : Inport
  pedAEBfullAborArb : Inport  Port=2
  pedAEBfullFuncImpAborCond : Inport  Port=3
  latBrkManeuAborCond : Inport  Port=4
  Compare To Constant{,1..3} (4 alike) : simulink/Logic and Bit Operations/Compare To Constant  relop===  const=TRUE  OutDataTypeStr=boolean
  Logical Operator : Logic  Operator=OR  Inputs=4  AllPortsSameDT=off  OutDataTypeStr=boolean
  ActiveStateAborCondFulfilled : Outport
  wires:
  Compare To Constant3/1 -> Logical Operator/4
  latBrkManeuAborCond/1 -> Compare To Constant3/1
  pedAEBfullAborCondStandstill/1 -> Compare To Constant/1
  pedAEBfullAborArb/1 -> Compare To Constant1/1
  pedAEBfullFuncImpAborCond/1 -> Compare To Constant2/1
  Compare To Constant1/1 -> Logical Operator/2
  Compare To Constant/1 -> Logical Operator/1
  Compare To Constant2/1 -> Logical Operator/3
  Logical Operator/1 -> ActiveStateAborCondFulfilled/1

== system TosAeb/FcwAebJa_Step_sys/ALG_AEBSystem/ALG_AEBController/AEB_StateMachineCalcApp/LatAEBStateMachine/pedPreWarningStateMachine/AEBFuncFaultMap ==
  FCW_AEB_VehFctSts : Inport
  Compare To Constant : simulink/Logic and Bit Operations/Compare To Constant  relop===  const=CN_VSC_FCW_AEB_Error  OutDataTypeStr=boolean
  Fault : Outport
  wires:
  FCW_AEB_VehFctSts/1 -> Compare To Constant/1
  Compare To Constant/1 -> Fault/1

== system TosAeb/FcwAebJa_Step_sys/ALG_AEBSystem/ALG_AEBController/AEB_StateMachineCalcApp/LatAEBStateMachine/pedPreWarningStateMachine/ActivationCondCalc ==
  pedPreWarningSuppCond : Inport
  pedPreWarningSuppArb : Inport  Port=2
  pedPreWarningFuncImpSuppCond : Inport  Port=3
  Compare To Constant : simulink/Logic and Bit Operations/Compare To Constant  relop===  const=FALSE  OutDataTypeStr=boolean
  Compare To Constant1 : simulink/Logic and Bit Operations/Compare To Constant  relop===  const=FALSE  OutDataTypeStr=boolean
  Compare To Constant2 : simulink/Logic and Bit Operations/Compare To Constant  relop===  const=FALSE  OutDataTypeStr=boolean
  Logical Operator : Logic  Inputs=3  AllPortsSameDT=off  OutDataTypeStr=boolean
  ActivationCondFulfilled : Outport
  wires:
  pedPreWarningSuppCond/1 -> Compare To Constant/1
  pedPreWarningSuppArb/1 -> Compare To Constant1/1
  pedPreWarningFuncImpSuppCond/1 -> Compare To Constant2/1
  Compare To Constant1/1 -> Logical Operator/2
  Compare To Constant/1 -> Logical Operator/1
  Compare To Constant2/1 -> Logical Operator/3
  Logical Operator/1 -> ActivationCondFulfilled/1

== system TosAeb/FcwAebJa_Step_sys/ALG_AEBSystem/ALG_AEBController/AEB_StateMachineCalcApp/LatAEBStateMachine/pedPreWarningStateMachine/ActiveStateAborCondCalc ==
  pedPreWarningAborCond : Inport
  pedPreWarningAborArb : Inport  Port=2
  pedPreWarningFuncImpAborCond : Inport  Port=3
  Compare To Constant : simulink/Logic and Bit Operations/Compare To Constant  relop===  const=TRUE  OutDataTypeStr=boolean
  Compare To Constant1 : simulink/Logic and Bit Operations/Compare To Constant  relop===  const=TRUE  OutDataTypeStr=boolean
  Compare To Constant2 : simulink/Logic and Bit Operations/Compare To Constant  relop===  const=TRUE  OutDataTypeStr=boolean
  Logical Operator : Logic  Operator=OR  Inputs=3  AllPortsSameDT=off  OutDataTypeStr=boolean
  ActiveStateAborCondFulfilled : Outport
  wires:
  Logical Operator/1 -> ActiveStateAborCondFulfilled/1
  Compare To Constant2/1 -> Logical Operator/3
  Compare To Constant/1 -> Logical Operator/1
  Compare To Constant1/1 -> Logical Operator/2
  pedPreWarningFuncImpAborCond/1 -> Compare To Constant2/1
  pedPreWarningAborArb/1 -> Compare To Constant1/1
  pedPreWarningAborCond/1 -> Compare To Constant/1

== system TosAeb/FcwAebJa_Step_sys/ALG_AEBSystem/ALG_AEBController/AEB_StateMachineCalcApp/LatAEBStateMachine/pedPrefillStateMachine/AEBFuncFaultMap ==
  FCW_AEB_VehFctSts : Inport
  Compare To Constant : simulink/Logic and Bit Operations/Compare To Constant  relop===  const=CN_VSC_FCW_AEB_Error  OutDataTypeStr=boolean
  Fault : Outport
  wires:
  FCW_AEB_VehFctSts/1 -> Compare To Constant/1
  Compare To Constant/1 -> Fault/1
